simulink model "vegas01_4tap_1024ch_250mhz_r1"  (Simulink 7.6)
settings: Solver=ode45  StartTime=0.0  StopTime=128000  RelTol=1e-3
undefined symbols (not defined in the file): ROACH
libraries: xbsIndex_r4 x12350, monroe_library x84, xbsReplacements_r4 x36, xps_library x30, casper_library_misc x24, dspsigops x16
(part 3 of 103)

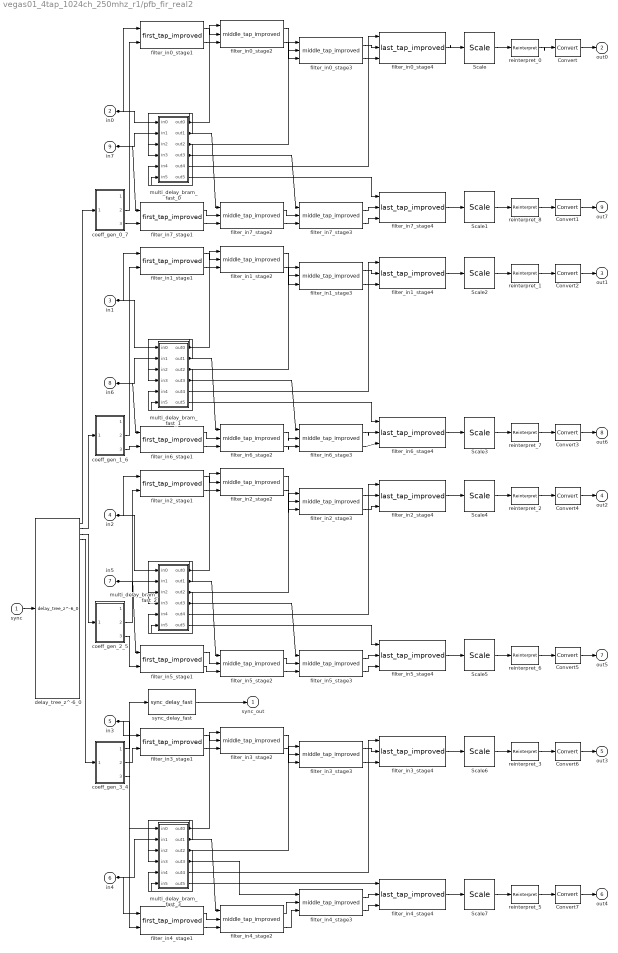
== system vegas01_4tap_1024ch_250mhz_r1/pfb_fir_real2 ==
  sync : Inport
  in0 : Inport  Port=2
  in1 : Inport  Port=3
  in2 : Inport  Port=4
  in3 : Inport  Port=5
  in4 : Inport  Port=6
  in5 : Inport  Port=7
  in6 : Inport  Port=8
  in7 : Inport  Port=9
  Convert{,1..7} (8 alike) : xbsIndex_r4/Convert  arith_type="Signed  (2's comp)"  n_bits=18  bin_pt=17  quantization=Truncate  overflow=Wrap  en=off  latency=1  dbl_ovrd=off  pipeline=off  block_type=convert
  Scale{,1..7} (8 alike) : xbsIndex_r4/Scale  scale_factor=-2  dbl_ovrd=off  block_type=scale
  coeff_gen_0_7 : Subsystem
  coeff_gen_1_6 : Subsystem
  coeff_gen_2_5 : Subsystem
  coeff_gen_3_4 : Subsystem
  delay_tree_z^-6_0 : monroe_library/delay_tree_z^-6_0  SystemSampleTime=-1
  filter_in0_stage1 : monroe_library/first_tap_improved  SystemSampleTime=-1  data_bin_pt=7  coeff_bit_width=18
  filter_in0_stage2 : monroe_library/middle_tap_improved  SystemSampleTime=-1  data_bin_pt=7  coeff_bit_width=18  stage_num=2
  filter_in0_stage3 : monroe_library/middle_tap_improved  SystemSampleTime=-1  data_bin_pt=7  coeff_bit_width=18  stage_num=3
  filter_in0_stage4 : monroe_library/last_tap_improved  SystemSampleTime=-1  data_bin_pt=7  coeff_bin_pt=17  stage_num=4
  filter_in1_stage1 : monroe_library/first_tap_improved  SystemSampleTime=-1  data_bin_pt=7  coeff_bit_width=18
  filter_in1_stage2 : monroe_library/middle_tap_improved  SystemSampleTime=-1  data_bin_pt=7  coeff_bit_width=18  stage_num=2
  filter_in1_stage3 : monroe_library/middle_tap_improved  SystemSampleTime=-1  data_bin_pt=7  coeff_bit_width=18  stage_num=3
  filter_in1_stage4 : monroe_library/last_tap_improved  SystemSampleTime=-1  data_bin_pt=7  coeff_bin_pt=17  stage_num=4
  filter_in2_stage1 : monroe_library/first_tap_improved  SystemSampleTime=-1  data_bin_pt=7  coeff_bit_width=18
  filter_in2_stage2 : monroe_library/middle_tap_improved  SystemSampleTime=-1  data_bin_pt=7  coeff_bit_width=18  stage_num=2
  filter_in2_stage3 : monroe_library/middle_tap_improved  SystemSampleTime=-1  data_bin_pt=7  coeff_bit_width=18  stage_num=3
  filter_in2_stage4 : monroe_library/last_tap_improved  SystemSampleTime=-1  data_bin_pt=7  coeff_bin_pt=17  stage_num=4
  filter_in3_stage1 : monroe_library/first_tap_improved  SystemSampleTime=-1  data_bin_pt=7  coeff_bit_width=18
  filter_in3_stage2 : monroe_library/middle_tap_improved  SystemSampleTime=-1  data_bin_pt=7  coeff_bit_width=18  stage_num=2
  filter_in3_stage3 : monroe_library/middle_tap_improved  SystemSampleTime=-1  data_bin_pt=7  coeff_bit_width=18  stage_num=3
  filter_in3_stage4 : monroe_library/last_tap_improved  SystemSampleTime=-1  data_bin_pt=7  coeff_bin_pt=17  stage_num=4
  filter_in4_stage1 : monroe_library/first_tap_improved  SystemSampleTime=-1  data_bin_pt=7  coeff_bit_width=18
  filter_in4_stage2 : monroe_library/middle_tap_improved  SystemSampleTime=-1  data_bin_pt=7  coeff_bit_width=18  stage_num=2
  filter_in4_stage3 : monroe_library/middle_tap_improved  SystemSampleTime=-1  data_bin_pt=7  coeff_bit_width=18  stage_num=3
  filter_in4_stage4 : monroe_library/last_tap_improved  SystemSampleTime=-1  data_bin_pt=7  coeff_bin_pt=17  stage_num=4
  filter_in5_stage1 : monroe_library/first_tap_improved  SystemSampleTime=-1  data_bin_pt=7  coeff_bit_width=18
  filter_in5_stage2 : monroe_library/middle_tap_improved  SystemSampleTime=-1  data_bin_pt=7  coeff_bit_width=18  stage_num=2
  filter_in5_stage3 : monroe_library/middle_tap_improved  SystemSampleTime=-1  data_bin_pt=7  coeff_bit_width=18  stage_num=3
  filter_in5_stage4 : monroe_library/last_tap_improved  SystemSampleTime=-1  data_bin_pt=7  coeff_bin_pt=17  stage_num=4
  filter_in6_stage1 : monroe_library/first_tap_improved  SystemSampleTime=-1  data_bin_pt=7  coeff_bit_width=18
  filter_in6_stage2 : monroe_library/middle_tap_improved  SystemSampleTime=-1  data_bin_pt=7  coeff_bit_width=18  stage_num=2
  filter_in6_stage3 : monroe_library/middle_tap_improved  SystemSampleTime=-1  data_bin_pt=7  coeff_bit_width=18  stage_num=3
  filter_in6_stage4 : monroe_library/last_tap_improved  SystemSampleTime=-1  data_bin_pt=7  coeff_bin_pt=17  stage_num=4
  filter_in7_stage1 : monroe_library/first_tap_improved  SystemSampleTime=-1  data_bin_pt=7  coeff_bit_width=18
  filter_in7_stage2 : monroe_library/middle_tap_improved  SystemSampleTime=-1  data_bin_pt=7  coeff_bit_width=18  stage_num=2
  filter_in7_stage3 : monroe_library/middle_tap_improved  SystemSampleTime=-1  data_bin_pt=7  coeff_bit_width=18  stage_num=3
  filter_in7_stage4 : monroe_library/last_tap_improved  SystemSampleTime=-1  data_bin_pt=7  coeff_bin_pt=17  stage_num=4
  multi_delay_bram_fast_0 : Subsystem
  multi_delay_bram_fast_1 : Subsystem
  multi_delay_bram_fast_2 : Subsystem
  multi_delay_bram_fast_3 : Subsystem
  reinterpret_{0..3,5..8} (8 alike) : xbsIndex_r4/Reinterpret  force_arith_type=on  arith_type="Signed  (2's comp)"  force_bin_pt=on  bin_pt=24  block_type=reinterpret
      settings: infoedit="Changes signal type without altering the binary representation.   You can changed the signal between signed and unsigned, and relocate the binary point.<br><br>Hardware notes: In hardware this block costs nothing.<br><br>Example:  Suppose the input is 6 bits wide, signed, with 2 fractional bits, and the output is forced to unsigned with 0 fractional bits.  Then an input of -2.0 (1110.00 in binary 2's complement) becomes an output of 56 (111000 in binary)."
  sync_delay_fast : monroe_library/sync_delay_fast  SystemSampleTime=-1  delay_len=1031
      link override Constant2: opselect=C  inp2=PCIN>>17  inp1=P  carry=CIN
  sync_out : Outport
  out0 : Outport  Port=2
  out1 : Outport  Port=3
  out2 : Outport  Port=4
  out3 : Outport  Port=5
  out4 : Outport  Port=6
  out5 : Outport  Port=7
  out6 : Outport  Port=8
  out7 : Outport  Port=9
  wires:
  in0/1 -> multi_delay_bram_fast_0/1(in0), filter_in0_stage1/1
  in7/1 -> multi_delay_bram_fast_0/2(in1), filter_in7_stage1/1
  multi_delay_bram_fast_0/1(out0) -> filter_in0_stage2/1, multi_delay_bram_fast_0/3(in2)
  multi_delay_bram_fast_0/2(out1) -> filter_in7_stage2/1, multi_delay_bram_fast_0/4(in3)
  multi_delay_bram_fast_0/3(out2) -> filter_in0_stage3/1, multi_delay_bram_fast_0/5(in4)
  multi_delay_bram_fast_0/4(out3) -> filter_in7_stage3/1, multi_delay_bram_fast_0/6(in5)
  in1/1 -> multi_delay_bram_fast_1/1(in0), filter_in1_stage1/1
  in6/1 -> multi_delay_bram_fast_1/2(in1), filter_in6_stage1/1
  multi_delay_bram_fast_1/1(out0) -> filter_in1_stage2/1, multi_delay_bram_fast_1/3(in2)
  multi_delay_bram_fast_1/2(out1) -> filter_in6_stage2/1, multi_delay_bram_fast_1/4(in3)
  multi_delay_bram_fast_1/3(out2) -> filter_in1_stage3/1, multi_delay_bram_fast_1/5(in4)
  multi_delay_bram_fast_1/4(out3) -> filter_in6_stage3/1, multi_delay_bram_fast_1/6(in5)
  in2/1 -> multi_delay_bram_fast_2/1(in0), filter_in2_stage1/1
  in5/1 -> multi_delay_bram_fast_2/2(in1), filter_in5_stage1/1
  multi_delay_bram_fast_2/1(out0) -> filter_in2_stage2/1, multi_delay_bram_fast_2/3(in2)
  multi_delay_bram_fast_2/2(out1) -> filter_in5_stage2/1, multi_delay_bram_fast_2/4(in3)
  multi_delay_bram_fast_2/3(out2) -> filter_in2_stage3/1, multi_delay_bram_fast_2/5(in4)
  multi_delay_bram_fast_2/4(out3) -> filter_in5_stage3/1, multi_delay_bram_fast_2/6(in5)
  in3/1 -> multi_delay_bram_fast_3/1(in0), filter_in3_stage1/1
  in4/1 -> multi_delay_bram_fast_3/2(in1), filter_in4_stage1/1
  multi_delay_bram_fast_3/1(out0) -> filter_in3_stage2/1, multi_delay_bram_fast_3/3(in2)
  multi_delay_bram_fast_3/2(out1) -> filter_in4_stage2/1, multi_delay_bram_fast_3/4(in3)
  multi_delay_bram_fast_3/3(out2) -> filter_in3_stage3/1, multi_delay_bram_fast_3/5(in4)
  multi_delay_bram_fast_3/4(out3) -> filter_in4_stage3/1, multi_delay_bram_fast_3/6(in5)
  sync_delay_fast/1 -> sync_out/1
  coeff_gen_3_4/1(sync_out) -> sync_delay_fast/1
  Convert7/1 -> out4/1
  reinterpret_5/1 -> Convert7/1
  Convert6/1 -> out3/1
  reinterpret_3/1 -> Convert6/1
  Scale7/1 -> reinterpret_5/1
  Scale6/1 -> reinterpret_3/1
  filter_in4_stage4/1 -> Scale7/1
  filter_in3_stage4/1 -> Scale6/1
  filter_in4_stage3/2 -> filter_in4_stage4/3
  filter_in4_stage3/1 -> filter_in4_stage4/2
  multi_delay_bram_fast_3/6(out5) -> filter_in4_stage4/1
  filter_in3_stage3/2 -> filter_in3_stage4/3
  filter_in3_stage3/1 -> filter_in3_stage4/2
  multi_delay_bram_fast_3/5(out4) -> filter_in3_stage4/1
  filter_in4_stage2/2 -> filter_in4_stage3/3
  filter_in4_stage2/1 -> filter_in4_stage3/2
  filter_in3_stage2/2 -> filter_in3_stage3/3
  filter_in3_stage2/1 -> filter_in3_stage3/2
  filter_in4_stage1/2 -> filter_in4_stage2/3
  filter_in4_stage1/1 -> filter_in4_stage2/2
  filter_in3_stage1/2 -> filter_in3_stage2/3
  filter_in3_stage1/1 -> filter_in3_stage2/2
  coeff_gen_3_4/3(coeff_rev) -> filter_in4_stage1/2
  coeff_gen_3_4/2(coeff) -> filter_in3_stage1/2
  delay_tree_z^-6_0/4 -> coeff_gen_3_4/1(sync)
  Convert5/1 -> out5/1
  reinterpret_6/1 -> Convert5/1
  Convert4/1 -> out2/1
  reinterpret_2/1 -> Convert4/1
  Scale5/1 -> reinterpret_6/1
  Scale4/1 -> reinterpret_2/1
  filter_in5_stage4/1 -> Scale5/1
  filter_in2_stage4/1 -> Scale4/1
  filter_in5_stage3/2 -> filter_in5_stage4/3
  filter_in5_stage3/1 -> filter_in5_stage4/2
  multi_delay_bram_fast_2/6(out5) -> filter_in5_stage4/1
  filter_in2_stage3/2 -> filter_in2_stage4/3
  filter_in2_stage3/1 -> filter_in2_stage4/2
  multi_delay_bram_fast_2/5(out4) -> filter_in2_stage4/1
  filter_in5_stage2/2 -> filter_in5_stage3/3
  filter_in5_stage2/1 -> filter_in5_stage3/2
  filter_in2_stage2/2 -> filter_in2_stage3/3
  filter_in2_stage2/1 -> filter_in2_stage3/2
  filter_in5_stage1/2 -> filter_in5_stage2/3
  filter_in5_stage1/1 -> filter_in5_stage2/2
  filter_in2_stage1/2 -> filter_in2_stage2/3
  filter_in2_stage1/1 -> filter_in2_stage2/2
  coeff_gen_2_5/3(coeff_rev) -> filter_in5_stage1/2
  coeff_gen_2_5/2(coeff) -> filter_in2_stage1/2
  delay_tree_z^-6_0/3 -> coeff_gen_2_5/1(sync)
  Convert3/1 -> out6/1
  reinterpret_7/1 -> Convert3/1
  Convert2/1 -> out1/1
  reinterpret_1/1 -> Convert2/1
  Scale3/1 -> reinterpret_7/1
  Scale2/1 -> reinterpret_1/1
  filter_in6_stage4/1 -> Scale3/1
  filter_in1_stage4/1 -> Scale2/1
  filter_in6_stage3/2 -> filter_in6_stage4/3
  filter_in6_stage3/1 -> filter_in6_stage4/2
  multi_delay_bram_fast_1/6(out5) -> filter_in6_stage4/1
  filter_in1_stage3/2 -> filter_in1_stage4/3
  filter_in1_stage3/1 -> filter_in1_stage4/2
  multi_delay_bram_fast_1/5(out4) -> filter_in1_stage4/1
  filter_in6_stage2/2 -> filter_in6_stage3/3
  filter_in6_stage2/1 -> filter_in6_stage3/2
  filter_in1_stage2/2 -> filter_in1_stage3/3
  filter_in1_stage2/1 -> filter_in1_stage3/2
  filter_in6_stage1/2 -> filter_in6_stage2/3
  filter_in6_stage1/1 -> filter_in6_stage2/2
  filter_in1_stage1/2 -> filter_in1_stage2/3
  filter_in1_stage1/1 -> filter_in1_stage2/2
  coeff_gen_1_6/3(coeff_rev) -> filter_in6_stage1/2
  coeff_gen_1_6/2(coeff) -> filter_in1_stage1/2
  delay_tree_z^-6_0/2 -> coeff_gen_1_6/1(sync)
  Convert1/1 -> out7/1
  reinterpret_8/1 -> Convert1/1
  Convert/1 -> out0/1
  reinterpret_0/1 -> Convert/1
  Scale1/1 -> reinterpret_8/1
  Scale/1 -> reinterpret_0/1
  filter_in7_stage4/1 -> Scale1/1
  filter_in0_stage4/1 -> Scale/1
  filter_in7_stage3/2 -> filter_in7_stage4/3
  filter_in7_stage3/1 -> filter_in7_stage4/2
  multi_delay_bram_fast_0/6(out5) -> filter_in7_stage4/1
  filter_in0_stage3/2 -> filter_in0_stage4/3
  filter_in0_stage3/1 -> filter_in0_stage4/2
  multi_delay_bram_fast_0/5(out4) -> filter_in0_stage4/1
  filter_in7_stage2/2 -> filter_in7_stage3/3
  filter_in7_stage2/1 -> filter_in7_stage3/2
  filter_in0_stage2/2 -> filter_in0_stage3/3
  filter_in0_stage2/1 -> filter_in0_stage3/2
  filter_in7_stage1/2 -> filter_in7_stage2/3
  filter_in7_stage1/1 -> filter_in7_stage2/2
  filter_in0_stage1/2 -> filter_in0_stage2/3
  filter_in0_stage1/1 -> filter_in0_stage2/2
  coeff_gen_0_7/3(coeff_rev) -> filter_in7_stage1/2
  coeff_gen_0_7/2(coeff) -> filter_in0_stage1/2
  delay_tree_z^-6_0/1 -> coeff_gen_0_7/1(sync)
  sync/1 -> delay_tree_z^-6_0/1

== system vegas01_4tap_1024ch_250mhz_r1/reshaper ==
  note: reset will be tied to negative edge
      | of vacc
  note: narrower data width must go to port A of dual port ram
  rst : Inport
  valid : Inport  Port=2
  din : Inport  Port=3
  Constant : xbsIndex_r4/Constant  arith_type=Unsigned  const=0  n_bits=outwid  bin_pt=0  explicit_period=on  period=1  equ=P=C  opselect=C  inp2=PCIN>>17  opr=+  inp1=P  carry=CIN  dbl_ovrd=off  block_type=constant
      settings: block_version=11.4
  Constant2 : xbsIndex_r4/Constant  arith_type=Boolean  const=0  n_bits=outwid  bin_pt=0  explicit_period=on  period=1  equ=P=C  opselect=C  inp2=PCIN>>17  opr=+  inp1=P  carry=CIN  dbl_ovrd=off  block_type=constant
      settings: block_version=11.4
  Constant3 : xbsIndex_r4/Constant  arith_type=Unsigned  const=headlen  n_bits=l2headlen  bin_pt=0  explicit_period=on  period=1  equ=P=C  opselect=C  inp2=PCIN>>17  opr=+  inp1=P  carry=CIN  dbl_ovrd=off  block_type=constant
      settings: block_version=11.4
  Counter : xbsIndex_r4/Counter  cnt_type=Free Running  cnt_to=Inf  operation=Up  start_count=0  cnt_by_val=1  arith_type=Unsigned  n_bits=l2inlen  bin_pt=0  load_pin=off  rst=on  en=on  explicit_period=on  period=1  dbl_ovrd=off  use_behavioral_HDL=off  implementation=Fabric  block_type=counter
      settings: block_version=11.4
  Counter3 : xbsIndex_r4/Counter  cnt_type=Free Running  cnt_to=1023  operation=Up  start_count=0  cnt_by_val=1  arith_type=Unsigned  n_bits=l2headlen + 1  bin_pt=0  load_pin=off  rst=on  en=on  explicit_period=on  period=1  dbl_ovrd=off  use_behavioral_HDL=off  implementation=Fabric  use_rpm=on  block_type=counter
      settings: xl_area=[0, 0, 0, 0, 0, 0, 0]  block_version=9.1.01
  Delay : xbsIndex_r4/Delay  en=off  latency=1  dbl_ovrd=off  reg_retiming=off  block_type=delay
      settings: block_version=11.4
  Delay1 : xbsIndex_r4/Delay  en=off  latency=2  dbl_ovrd=off  reg_retiming=off  block_type=delay
      settings: block_version=11.4
  Delay2 : xbsIndex_r4/Delay  en=off  latency=2  dbl_ovrd=off  reg_retiming=off  block_type=delay
      settings: block_version=11.4
  Dual Port RAM : xbsIndex_r4/Dual Port RAM  depth=outlen  initVector=sin(pi*(0:outlen)/16)  distributed_mem=Block RAM  init_a=0  init_b=0  rst_a=off  rst_b=off  en_a=off  en_b=off  latency=2  write_mode_A=Read After Write  write_mode_B=Read After Write  dbl_ovrd=off  optimize=Speed  use_rpm=on  block_type=dpram
      settings: block_version=11.4
  Inverter : xbsIndex_r4/Inverter  en=off  latency=0  dbl_ovrd=off  block_type=inv
      settings: block_version=11.4
  Inverter1 : xbsIndex_r4/Inverter  en=off  latency=0  dbl_ovrd=off  block_type=inv
      settings: block_version=11.4
  Logical : xbsIndex_r4/Logical  logical_function=AND  inputs=2  en=off  latency=0  precision=Full  arith_type=Unsigned  n_bits=16  bin_pt=0  align_bp=on  dbl_ovrd=off  block_type=logical
      settings: block_version=11.4
  Logical1 : xbsIndex_r4/Logical  logical_function=AND  inputs=2  en=off  latency=0  precision=Full  arith_type=Unsigned  n_bits=16  bin_pt=0  align_bp=on  dbl_ovrd=off  block_type=logical
      settings: block_version=11.4
  MCode : xbsIndex_r4/MCode  mfname=reshaper_fsm.m  explicit_period=off  period=1  inputsTable={'boundInpExpr'=>['','','','',''],'inputs'=>['end_chunk','end_block','rst','valid_in','head_done']}  outputsTable={'outputs'=>['block_count_rst','chunk_count_rst','chunk_count_en','sel_rst','sel_en','header_valid','data_valid','st'],'suppressOut'=>['off','off','off','off','off','off','off','off']}  dbl_ovrd=off  enable_stdout=off  enable_debug=off  suppress_output=on  defparams={}  hide_port_list={}  block_type=mcode
      settings: block_version=11.4
  Relational : xbsIndex_r4/Relational  mode=a>=b  en=off  latency=0  dbl_ovrd=off  block_type=relational
  Terminator : Terminator
  block_counter : casper_library_misc/freeze_cntr  SystemSampleTime=-1  CounterBits=l2outlen
  block_done : xbsIndex_r4/Gateway Out  hdl_port=off  timing_constraint=None  locs_specified=off  LOCs={}  block_type=gatewayout
      settings: infoedit="Gateway out block.  Converts Xilinx fixed point inputs into ouputs of type Simulink integer, double, or fixed point.<br><br>Hardware notes:  In hardware these blocks become top level output ports or are discarded, depending on how they are configured."  block_version=11.4
  chunk_done_p : xbsIndex_r4/Gateway Out  hdl_port=off  timing_constraint=None  locs_specified=off  LOCs={}  block_type=gatewayout
      settings: infoedit="Gateway out block.  Converts Xilinx fixed point inputs into ouputs of type Simulink integer, double, or fixed point.<br><br>Hardware notes:  In hardware these blocks become top level output ports or are discarded, depending on how they are configured."  block_version=11.4
  chunkcounter : casper_library_misc/freeze_cntr  SystemSampleTime=-1  CounterBits=l2outchunk
  enable : xbsIndex_r4/Slice  nbits=l2headlen  boolean_output=off  mode=Lower Bit Location + Width  bit1=0  base1=LSB of Input  bit0=0  base0=LSB of Input  dbl_ovrd=off  block_type=slice
      settings: block_version=9.1.01
  head_done_p1 : xbsIndex_r4/Gateway Out  hdl_port=off  timing_constraint=None  locs_specified=off  LOCs={}  block_type=gatewayout
      settings: infoedit="Gateway out block.  Converts Xilinx fixed point inputs into ouputs of type Simulink integer, double, or fixed point.<br><br>Hardware notes:  In hardware these blocks become top level output ports or are discarded, depending on how they are configured."  block_version=11.4
  dout : Outport
  sel : Outport  Port=2
  header_valid_out : Outport  Port=3
  data_valid_out : Outport  Port=4
  st : Outport  Port=5
  wires:
  Logical1/1 -> Delay2/1
  MCode/7 -> Logical1/2
  Delay2/1 -> data_valid_out/1
  MCode/6 -> Delay1/1
  MCode/8 -> st/1
  [chunk_done] -> Inverter1/1
  Inverter1/1 -> Logical1/1
  [chunk_done] -> Inverter/1
  Inverter/1 -> Logical/1
  Logical/1 -> block_counter/1
  [blk_cnt_en] -> Logical/2
  Delay1/1 -> header_valid_out/1
  Delay/1 -> sel/1
  din/1 -> Dual Port RAM/5
  Counter/1 -> Dual Port RAM/4  "dinadddr"
  Constant2/1 -> Dual Port RAM/3
  Constant/1 -> Dual Port RAM/2
  block_counter/1 -> Dual Port RAM/1  "doutaddr"
  Dual Port RAM/2 -> Terminator/1
  Dual Port RAM/1 -> dout/1
  [valid] -> MCode/4
  [rst] -> MCode/3
  [head_done] -> MCode/5
  [block_done] -> MCode/2  "blockdone"
  [chunk_done] -> MCode/1  "chunkdone"
  MCode/4 -> [sel_cnt_rst]  "sel_cnt_rst"
  MCode/5 -> [sel_cnt_en]  "sel_cnt_en"
  Relational/1 -> head_done_p1/1, [head_done]  "headdone"
  enable/1 -> Delay/1
  [sel_cnt_en] -> Counter3/2
  Constant3/1 -> Relational/2
  Counter3/1 -> Relational/1, enable/1
  [sel_cnt_rst] -> Counter3/1
  MCode/2 -> [chunk_cnt_rst]  "chunk_cnt_rst"
  MCode/1 -> [blk_cnt_rst]  "blk_cnt_rst"
  MCode/3 -> [blk_cnt_en]  "chunk_count_nen"
  [blk_cnt_rst] -> block_counter/2  "blk_cnt_rst"
  block_counter/3 -> block_done/1, [block_done]  "block_dneone"
  [chunk_cnt_rst] -> chunkcounter/2  "chunk_cnt_rst"
  chunkcounter/3 -> chunk_done_p/1, [chunk_done]  "chunk_done"
  [blk_cnt_en] -> chunkcounter/1  "blk_cnt_en"
  valid/1 -> Dual Port RAM/6, [valid], Counter/2
  rst/1 -> [rst], Counter/1

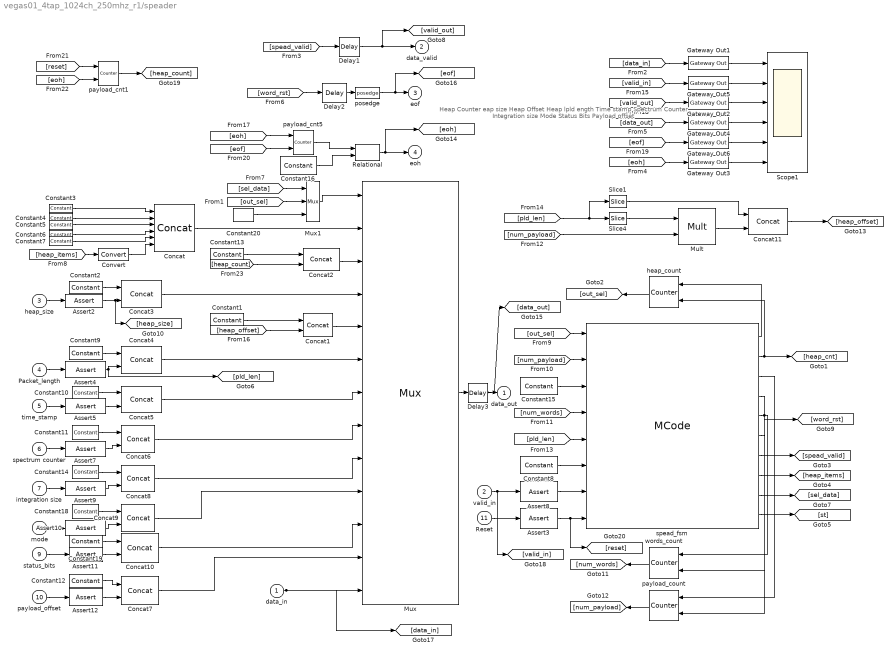
== system vegas01_4tap_1024ch_250mhz_r1/speader ==
  note: Heap Counter
      | eap size
      | Heap Offset
      | Heap lpld ength
      | Time stamp
      | Spectrum Counter
      | Integration size
      | Mode
      | Status Bits
      | Payload offset
  data_in : Inport
  valid_in : Inport  Port=2
  heap_size : Inport  Port=3
  Packet_length : Inport  Port=4
  time_stamp : Inport  Port=5
  spectrum counter : Inport  Port=6
  integration size : Inport  Port=7
  mode : Inport  Port=8
  status_bits : Inport  Port=9
  payload_offset : Inport  Port=10
  Reset : Inport  Port=11
  Assert{2,4..5,7,9..12} (8 alike) : xbsIndex_r4/Assert  assert_type=on  type_source=Explicitly  arith_type=Unsigned  n_bits=spead_lsw  bin_pt=0  assert_rate=off  rate_source=Explicitly  period=1  output_port=on  block_type=assert
      settings: block_version=11.4
  Assert3 : xbsIndex_r4/Assert  assert_type=on  type_source=Explicitly  arith_type=Boolean  n_bits=spead_lsw  bin_pt=0  assert_rate=on  rate_source=Explicitly  period=1  output_port=on  block_type=assert
      settings: block_version=11.4
  Assert8 : xbsIndex_r4/Assert  assert_type=on  type_source=Explicitly  arith_type=Boolean  n_bits=spead_lsw  bin_pt=0  assert_rate=on  rate_source=Explicitly  period=1  output_port=on  block_type=assert
      settings: block_version=11.4
  Concat : xbsIndex_r4/Concat  num_inputs=6  dbl_ovrd=off  block_type=concat
  Concat{1..11} (11 alike) : xbsIndex_r4/Concat  num_inputs=2  dbl_ovrd=off  block_type=concat
  Constant1 : xbsIndex_r4/Constant  arith_type=Unsigned  const=8388608+3  n_bits=spead_msw-spead_lsw  bin_pt=0  explicit_period=on  period=1  equ=P=C  opselect=C  inp2=PCIN>>17  opr=+  inp1=P  carry=CIN  dbl_ovrd=off  block_type=constant
      settings: block_version=11.4
  Constant10 : xbsIndex_r4/Constant  arith_type=Unsigned  const=8388608+32  n_bits=spead_msw-spead_lsw  bin_pt=0  explicit_period=on  period=1  equ=P=C  opselect=C  inp2=PCIN>>17  opr=+  inp1=P  carry=CIN  dbl_ovrd=off  block_type=constant
      settings: block_version=11.4
  Constant11 : xbsIndex_r4/Constant  arith_type=Unsigned  const=8388608+33  n_bits=spead_msw-spead_lsw  bin_pt=0  explicit_period=on  period=1  equ=P=C  opselect=C  inp2=PCIN>>17  opr=+  inp1=P  carry=CIN  dbl_ovrd=off  block_type=constant
      settings: block_version=11.4
  Constant12 : xbsIndex_r4/Constant  arith_type=Unsigned  const=37  n_bits=spead_msw-spead_lsw  bin_pt=0  explicit_period=on  period=1  equ=P=C  opselect=C  inp2=PCIN>>17  opr=+  inp1=P  carry=CIN  dbl_ovrd=off  block_type=constant
      settings: block_version=11.4
  Constant13 : xbsIndex_r4/Constant  arith_type=Unsigned  const=8388608+1  n_bits=spead_msw-spead_lsw  bin_pt=0  explicit_period=on  period=1  equ=P=C  opselect=C  inp2=PCIN>>17  opr=+  inp1=P  carry=CIN  dbl_ovrd=off  block_type=constant
      settings: block_version=11.4
  Constant14 : xbsIndex_r4/Constant  arith_type=Unsigned  const=8388608+34  n_bits=spead_msw-spead_lsw  bin_pt=0  explicit_period=on  period=1  equ=P=C  opselect=C  inp2=PCIN>>17  opr=+  inp1=P  carry=CIN  dbl_ovrd=off  block_type=constant
      settings: block_version=11.4
  Constant15 : xbsIndex_r4/Constant  arith_type=Unsigned  const=num_items+5  n_bits=8  bin_pt=0  explicit_period=on  period=1  equ=P=C  opselect=C  inp2=PCIN>>17  opr=+  inp1=P  carry=CIN  dbl_ovrd=off  block_type=constant
      settings: block_version=11.4
  Constant16 : xbsIndex_r4/Constant  arith_type=Unsigned  const=num_payloads  n_bits=4  bin_pt=0  explicit_period=on  period=1  equ=P=C  opselect=C  inp2=PCIN>>17  opr=+  inp1=P  carry=CIN  dbl_ovrd=off  block_type=constant
      settings: block_version=11.4
  Constant18 : xbsIndex_r4/Constant  arith_type=Unsigned  const=8388608+35  n_bits=spead_msw-spead_lsw  bin_pt=0  explicit_period=on  period=1  equ=P=C  opselect=C  inp2=PCIN>>17  opr=+  inp1=P  carry=CIN  dbl_ovrd=off  block_type=constant
      settings: block_version=11.4
  Constant19 : xbsIndex_r4/Constant  arith_type=Unsigned  const=8388608+36  n_bits=spead_msw-spead_lsw  bin_pt=0  explicit_period=on  period=1  equ=P=C  opselect=C  inp2=PCIN>>17  opr=+  inp1=P  carry=CIN  dbl_ovrd=off  block_type=constant
      settings: block_version=11.4
  Constant2 : xbsIndex_r4/Constant  arith_type=Unsigned  const=8388608+2  n_bits=spead_msw-spead_lsw  bin_pt=0  explicit_period=on  period=1  equ=P=C  opselect=C  inp2=PCIN>>17  opr=+  inp1=P  carry=CIN  dbl_ovrd=off  block_type=constant
      settings: block_version=11.4
  Constant20 : xbsIndex_r4/Constant  arith_type=Unsigned  const=num_items+5  n_bits=4  bin_pt=0  explicit_period=on  period=1  equ=P=C  opselect=C  inp2=PCIN>>17  opr=+  inp1=P  carry=CIN  dbl_ovrd=off  block_type=constant
      settings: block_version=11.4
  Constant3 : xbsIndex_r4/Constant  arith_type=Unsigned  const=83  n_bits=8  bin_pt=0  explicit_period=on  period=1  equ=P=C  opselect=C  inp2=PCIN>>17  opr=+  inp1=P  carry=CIN  dbl_ovrd=off  block_type=constant
      settings: block_version=11.4
  Constant4 : xbsIndex_r4/Constant  arith_type=Unsigned  const=4  n_bits=8  bin_pt=0  explicit_period=on  period=1  equ=P=C  opselect=C  inp2=PCIN>>17  opr=+  inp1=P  carry=CIN  dbl_ovrd=off  block_type=constant
      settings: block_version=11.4
  Constant5 : xbsIndex_r4/Constant  arith_type=Unsigned  const=3  n_bits=8  bin_pt=0  explicit_period=on  period=1  equ=P=C  opselect=C  inp2=PCIN>>17  opr=+  inp1=P  carry=CIN  dbl_ovrd=off  block_type=constant
      settings: block_version=11.4
  Constant6 : xbsIndex_r4/Constant  arith_type=Unsigned  const=5  n_bits=8  bin_pt=0  explicit_period=on  period=1  equ=P=C  opselect=C  inp2=PCIN>>17  opr=+  inp1=P  carry=CIN  dbl_ovrd=off  block_type=constant
      settings: block_version=11.4
  Constant7 : xbsIndex_r4/Constant  arith_type=Unsigned  const=0  n_bits=16  bin_pt=0  explicit_period=on  period=1  equ=P=C  opselect=C  inp2=PCIN>>17  opr=+  inp1=P  carry=CIN  dbl_ovrd=off  block_type=constant
      settings: block_version=11.4
  Constant8 : xbsIndex_r4/Constant  arith_type=Unsigned  const=num_payloads  n_bits=8  bin_pt=0  explicit_period=on  period=1  equ=P=C  opselect=C  inp2=PCIN>>17  opr=+  inp1=P  carry=CIN  dbl_ovrd=off  block_type=constant
      settings: block_version=11.4
  Constant9 : xbsIndex_r4/Constant  arith_type=Unsigned  const=8388608+4  n_bits=spead_msw-spead_lsw  bin_pt=0  explicit_period=on  period=1  equ=P=C  opselect=C  inp2=PCIN>>17  opr=+  inp1=P  carry=CIN  dbl_ovrd=off  block_type=constant
      settings: block_version=11.4
  Convert : xbsIndex_r4/Convert  arith_type=Unsigned  n_bits=16  bin_pt=0  quantization=Truncate  overflow=Wrap  en=off  latency=0  dbl_ovrd=off  pipeline=off  block_type=convert
      settings: block_version=11.4
  Delay1 : xbsIndex_r4/Delay  en=off  latency=1  dbl_ovrd=off  reg_retiming=on  block_type=delay
      settings: block_version=11.4
  Delay2 : xbsIndex_r4/Delay  en=off  latency=0  dbl_ovrd=off  reg_retiming=on  block_type=delay
      settings: block_version=11.4
  Delay3 : xbsIndex_r4/Delay  en=off  latency=1  dbl_ovrd=off  reg_retiming=on  block_type=delay
      settings: block_version=11.4
  Gateway Out{1..6} (6 alike) : xbsIndex_r4/Gateway Out  hdl_port=on  timing_constraint=None  locs_specified=off  LOCs={}  block_type=gatewayout
      settings: infoedit="Gateway out block.  Converts Xilinx fixed point inputs into ouputs of type Simulink integer, double, or fixed point.<br><br>Hardware notes:  In hardware these blocks become top level output ports or are discarded, depending on how they are configured."  block_version=11.4
  Mult : xbsIndex_r4/Mult  precision=User Defined  arith_type=Unsigned  n_bits=25  bin_pt=0  quantization=Truncate  overflow=Wrap  en=off  latency=3  dbl_ovrd=off  use_behavioral_HDL=off  opt=Speed  use_embedded=on  optimum_pipeline=off  pipeline=on  use_rpm=on  placement_style=Triangular  block_type=mult
      settings: block_version=11.4
  Mux : xbsIndex_r4/Mux  inputs=12  en=off  latency=0  precision=User Defined  arith_type=Unsigned  n_bits=64  bin_pt=0  quantization=Truncate  overflow=Wrap  dbl_ovrd=off  block_type=mux
      settings: block_version=11.4
  Mux1 : xbsIndex_r4/Mux  inputs=2  en=off  latency=0  precision=Full  arith_type="Signed  (2's comp)"  n_bits=8  bin_pt=2  quantization=Truncate  overflow=Wrap  dbl_ovrd=off  block_type=mux
      settings: block_version=11.4
  Relational : xbsIndex_r4/Relational  mode=a=b  en=off  latency=0  dbl_ovrd=off  block_type=relational
  Scope1 : Scope  NumInputPorts=6
      settings: TimeRange=1000  YMin=0~0~0~0~0~0  YMax=40~1~1~1e+19~1~20  SaveName=ScopeData2  MaxDataPoints=10000
  Slice1 : xbsIndex_r4/Slice  nbits=15  boolean_output=off  mode=Upper Bit Location + Width  bit1=0  base1=MSB of Input  bit0=0  base0=LSB of Input  dbl_ovrd=off  block_type=slice
      settings: block_version=10.1.3
  Slice4 : xbsIndex_r4/Slice  nbits=25  boolean_output=off  mode=Lower Bit Location + Width  bit1=-1  base1=MSB of Input  bit0=0  base0=LSB of Input  dbl_ovrd=off  block_type=slice
      settings: block_version=10.1.3
  heap_count : xbsIndex_r4/Counter  cnt_type=Free Running  cnt_to=Inf  operation=Up  start_count=0  cnt_by_val=1  arith_type=Unsigned  n_bits=4  bin_pt=0  load_pin=off  rst=on  en=on  explicit_period=on  period=1  dbl_ovrd=off  use_behavioral_HDL=off  implementation=Fabric  block_type=counter
      settings: block_version=11.4
  payload_cnt1 : xbsIndex_r4/Counter  cnt_type=Free Running  cnt_to=0  operation=Up  start_count=1024  cnt_by_val=1  arith_type=Unsigned  n_bits=40  bin_pt=0  load_pin=off  rst=on  en=on  explicit_period=on  period=1  dbl_ovrd=off  use_behavioral_HDL=off  implementation=Fabric  use_rpm=on  block_type=counter
      settings: xl_area=[0, 0, 0, 0, 0, 0, 0]
  payload_cnt5 : xbsIndex_r4/Counter  cnt_type=Free Running  cnt_to=0  operation=Up  start_count=0  cnt_by_val=1  arith_type=Unsigned  n_bits=4  bin_pt=0  load_pin=off  rst=on  en=on  explicit_period=on  period=1  dbl_ovrd=off  use_behavioral_HDL=off  implementation=Fabric  use_rpm=on  block_type=counter
      settings: xl_area=[0, 0, 0, 0, 0, 0, 0]
  payload_count : xbsIndex_r4/Counter  cnt_type=Free Running  cnt_to=Inf  operation=Up  start_count=0  cnt_by_val=1  arith_type=Unsigned  n_bits=8  bin_pt=0  load_pin=off  rst=on  en=on  explicit_period=on  period=1  dbl_ovrd=off  use_behavioral_HDL=off  implementation=Fabric  block_type=counter
      settings: block_version=11.4
  posedge : casper_library_misc/posedge  Tag=Rising Edge Detector  SystemSampleTime=-1
  spead_fsm : xbsIndex_r4/MCode  mfname=spead_fsm  explicit_period=on  period=1  inputsTable={'boundInpExpr'=>['','','','5','','','','',''],'inputs'=>['head_count','num_payload_sent','total_header_len','min_header_len','num_words_sent','payload_len','total_num_payloads','data_valid_in','rst']}  outputsTable={'outputs'=>['head_count_rst','head_count_en','num_payload_rst','num_payload_en','words_sent_rst','words_sent_en','tge_valid','heap_elements','select_data','st'],'suppressOut'=>['off','off','off','off','off','off','off','off','off','off']}  dbl_ovrd=off  enable_stdout=off  enable_debug=off  suppress_output=on  defparams={'min_header_len',5}  hide_port_list={}  block_type=mcode
      settings: block_version=11.4
  words_count : xbsIndex_r4/Counter  cnt_type=Free Running  cnt_to=Inf  operation=Up  start_count=0  cnt_by_val=8  arith_type=Unsigned  n_bits=15  bin_pt=0  load_pin=off  rst=on  en=on  explicit_period=on  period=1  dbl_ovrd=off  use_behavioral_HDL=off  implementation=Fabric  block_type=counter
      settings: block_version=11.4
  data_out : Outport
  data_valid : Outport  Port=2
  eof : Outport  Port=3
  eoh : Outport  Port=4
  wires:
  Assert12/1 -> Concat7/2
  payload_offset/1 -> Assert12/1
  Assert11/1 -> Concat10/2
  status_bits/1 -> Assert11/1
  Constant19/1 -> Concat10/1
  Assert10/1 -> Concat9/2
  mode/1 -> Assert10/1
  Constant18/1 -> Concat9/1
  Concat4/1 -> Mux/6
  Concat3/1 -> Mux/4
  Concat/1 -> Mux/2
  Constant9/1 -> Concat4/1
  Constant7/1 -> Concat/5
  Constant6/1 -> Concat/4
  Constant5/1 -> Concat/3
  Constant4/1 -> Concat/2
  Constant3/1 -> Concat/1
  Constant2/1 -> Concat3/1
  Constant13/1 -> Concat2/1
  Constant12/1 -> Concat7/1
  Constant10/1 -> Concat5/1
  data_in/1 -> [data_in], Mux/13
  time_stamp/1 -> Assert5/1
  heap_size/1 -> Assert2/1
  [heap_count] -> Concat2/2
  Assert2/1 -> Concat3/2, [heap_size]
  Assert4/1 -> Concat4/2, [pld_len]
  Assert5/1 -> Concat5/2
  Concat2/1 -> Mux/3
  [spead_valid] -> Delay1/1
  Packet_length/1 -> Assert4/1
  [valid_out] -> Gateway Out2/1
  Delay1/1 -> data_valid/1, [valid_out]
  [eof] -> Gateway Out6/1
  Constant1/1 -> Concat1/1
  Concat1/1 -> Mux/5
  Concat7/1 -> Mux/12
  Concat10/1 -> Mux/11
  Concat9/1 -> Mux/10
  spectrum counter/1 -> Assert7/1
  Assert7/1 -> Concat6/2
  Constant11/1 -> Concat6/1
  Concat6/1 -> Mux/8
  Concat5/1 -> Mux/7
  integration size/1 -> Assert9/1
  Assert9/1 -> Concat8/2
  Constant14/1 -> Concat8/1
  Concat8/1 -> Mux/9
  Gateway Out2/1 -> Scope1/3
  Gateway Out6/1 -> Scope1/5
  spead_fsm/5 -> words_count/1
  spead_fsm/6 -> words_count/2
  spead_fsm/3 -> payload_count/1
  spead_fsm/4 -> payload_count/2, [word_rst]
  spead_fsm/1 -> heap_count/1
  spead_fsm/2 -> heap_count/2, [heap_cnt]
  spead_fsm/7 -> [spead_valid]
  spead_fsm/8 -> [heap_items]
  valid_in/1 -> Assert8/1, [valid_in]
  [heap_offset] -> Concat1/2
  Reset/1 -> Assert3/1
  [data_in] -> Gateway Out1/1
  Gateway Out1/1 -> Scope1/1
  Gateway Out3/1 -> Scope1/6
  Gateway Out4/1 -> Scope1/4
  [data_out] -> Gateway Out4/1
  Constant20/1 -> Mux1/3
  Mux1/1 -> Mux/1
  spead_fsm/10 -> [st]
  spead_fsm/9 -> [sel_data]
  [heap_items] -> Convert/1
  Convert/1 -> Concat/6
  words_count/1 -> [num_words]
  payload_count/1 -> [num_payload]
  heap_count/1 -> [out_sel]
  [out_sel] -> spead_fsm/1
  [num_payload] -> spead_fsm/2
  [valid_in] -> Gateway Out5/1
  Gateway Out5/1 -> Scope1/2
  posedge/1 -> eof/1, [eof]
  [word_rst] -> Delay2/1
  Assert3/1 -> spead_fsm/8, [reset]
  Assert8/1 -> spead_fsm/7
  Constant8/1 -> spead_fsm/6
  [pld_len] -> spead_fsm/5
  [num_words] -> spead_fsm/4
  Constant15/1 -> spead_fsm/3
  Delay2/1 -> posedge/1
  [num_payload] -> Mult/2
  [pld_len] -> Slice4/1, Slice1/1
  Delay3/1 -> data_out/1, [data_out]
  Mux/1 -> Delay3/1
  [out_sel] -> Mux1/2
  [sel_data] -> Mux1/1
  [eoh] -> Gateway Out3/1
  [eoh] -> payload_cnt5/1
  [eof] -> payload_cnt5/2
  Relational/1 -> eoh/1, [eoh]
  Constant16/1 -> Relational/2
  payload_cnt5/1 -> Relational/1
  payload_cnt1/1 -> [heap_count]
  [reset] -> payload_cnt1/1
  [eoh] -> payload_cnt1/2
  Concat11/1 -> [heap_offset]
  Mult/1 -> Concat11/2
  Slice4/1 -> Mult/1
  Slice1/1 -> Concat11/1
